simulink model "Complete_Model"  (R2023a)
settings: Solver=VariableStepAuto  StartTime=0.0  StopTime=22  MinStep=5e-5  RelTol=1e-3
values from neighbouring files (symbols this model uses):
  Cbus = 80e-3*n_wt_agg*u^2    (= 1.33333333333)    [param.m]
  D = 1e-3    (= 0.001)    [param.m]
  f = 50    [param.m]
  Fs = Vs * sqrt(2/3)/(2*pi*f)    [param.m]
  fsw = 4e3    (= 4000)    [param.m]
  Idr_rated = sqrt(2)*Is    [param.m]
  J = 127*n_wt_agg    (= 19050)    [param.m]
  ki_id = (wni^2)*Lr*sigma    (= 3.27733001332)    [param.m]
  ki_idg = (wnig^2)*Lg    (= 0.263189450696)    [param.m]
  ki_iq = ki_id    (= 3.27733001332)    [param.m]
  ki_iqg = ki_idg    (= 0.263189450696)    [param.m]
  ki_v = -300000*n_wt_agg    (= -45000000)    [param.m]
  Kopt = ((0.5*rho*pi*(Radius^5)*Cp_max)/(lambda_opt^3))    (= 296454.198186)    [param.m]
  kp_id = (2*wni*sigma*Lr) - Rr    (= 0.00384733333333)    [param.m]
  kp_idg = ((2*wnig*Lg)-Rg)    (= 0.00167538274858)    [param.m]
  kp_iq = kp_id    (= 0.00384733333333)    [param.m]
  kp_iqg = kp_idg    (= 0.00167538274858)    [param.m]
  kp_v = -1000*n_wt_agg    (= -150000)    [param.m]
  Kpg = 1/(1.5*Vr*sqrt(2/3))    [param.m]
  Kqg = -Kpg    [param.m]
  lambda_opt = 7.2    [param.m]
  Lg = 400e-6 /n_wt_agg    (= 2.66666666667e-06)    [param.m]
  Lm = 2.5e-3 /n_wt_agg    (= 1.66666666667e-05)    [param.m]
  Lr = Lm + Lsi    (= 1.72466666667e-05)    [param.m]
  Ls = Lm + Lsi    (= 1.72466666667e-05)    [param.m]
  Lsi = 0.087e-3 /n_wt_agg    (= 5.8e-07)    [param.m]
  N = 100    [param.m]
  n_wt_agg = 150    [param.m]
  p = 2    [param.m]
  Ps = 2e6*n_wt_agg    (= 300000000)    [param.m]
  Radius = 42    [param.m]
  Rg = 20e-6 /n_wt_agg    (= 1.33333333333e-07)    [param.m]
  rho = 1.225    [param.m]
  Rr = 2.9e-3 /n_wt_agg    (= 1.93333333333e-05)    [param.m]
  Rs = 2.6e-3 /n_wt_agg    (= 1.73333333333e-05)    [param.m]
  sigma = 1 - Lm^2 / (Ls*Lr)    (= 0.0661284179513)    [param.m]
  smax = 1/3    (= 0.333333333333)    [param.m]
  tab_lambda = [0.1:0.01:11.8]    [param.m]
  Vbus = 1150/u    (= 3450)    [param.m]
  Vs = 690    [param.m]
undefined symbols (not defined in the file or in its folder): Cp, D1, Delta, DiscIntNeverNeededParam, magnitude
libraries: spsSecondOrderFilterLib x133, spsThreePhaseVIMeasurementLib x58, spsPower3phInstantaneousLib x52, simulink x41, spsThreePhaseTransformerTwoWindingsLib x27, spsUniversalBridgeLib x22, spsPWMGenerator2LevelLib x22, spsSeriesRLCBranchLib x19, spsFirstOrderFilterLib x18, spsThreePhasePISectionLineLib x17, spsGroundLib x17, spsThreePhaseSeriesRLCBranchLib x17
(part 12 of 15)

== system Complete_Model/Equivalent Wind Park/300MW Eq WT10/Power Calculations/angle2 ==
  Vs_mes : Inport
  Demux : Demux  Outputs=2
  Simple PLL : Trigonometry  Operator=atan2
  abc->alpha/beta : Subsystem
  theta_g : Outport
  wires:
  Simple PLL/1 -> theta_g/1  "theta_g"
  Demux/2 -> Simple PLL/1
  Demux/1 -> Simple PLL/2
  abc->alpha/beta/1(alpha/beta) -> Demux/1
  Vs_mes/1 -> abc->alpha/beta/1(abc)

== system Complete_Model/Equivalent Wind Park/300MW Eq WT10/Rotor Side Control/D//Q->abc ==
  note: D/Q = alpha/beta en fait
  D/Q : Inport
  Fcn : Fcn  Expr=u[1]
  Fcn1 : Fcn  Expr=-(u[1]/2) + u[2]*(sqrt(3)/2)
  Fcn2 : Fcn  Expr=-(u[1]/2) - u[2]*(sqrt(3)/2)
  Mux : Mux  Inputs=3
  abc : Outport
  wires:
  Fcn/1 -> Mux/1
  Fcn2/1 -> Mux/3
  Mux/1 -> abc/1
  Fcn1/1 -> Mux/2
  D/Q/1 -> Fcn/1, Fcn1/1, Fcn2/1

== system Complete_Model/Equivalent Wind Park/300MW Eq WT10/Rotor Side Control/D//Q->d//q ==
  D/Q : Inport
  theta : Inport  Port=2
  Fcn : Fcn  Expr=u[1]*cos(u[3]) + u[2]*sin(u[3])
  Fcn1 : Fcn  Expr=-u[1]*sin(u[3]) + u[2]*cos(u[3])
  Mux : Mux  Inputs=2
  Mux1 : Mux  Inputs=2
  d/q : Outport
  wires:
  Fcn1/1 -> Mux/2
  Mux1/1 -> Fcn1/1, Fcn/1
  Fcn/1 -> Mux/1
  theta/1 -> Mux1/2
  D/Q/1 -> Mux1/1
  Mux/1 -> d/q/1

== system Complete_Model/Equivalent Wind Park/300MW Eq WT10/Rotor Side Control/MPPT ==
  omega_m : Inport
  Gain : Gain  Gain=1/N
  Gain1 : Gain  Gain=-Kopt
  Gain2 : Gain  Gain=1/N
  Gain3 : Gain  Gain=n_wt_agg
  Square : Math  Operator=square
  Tm,opt : Outport
  wires:
  Gain3/1 -> Tm,opt/1
  Gain2/1 -> Gain3/1
  Gain1/1 -> Gain2/1
  Square/1 -> Gain1/1
  Gain/1 -> Square/1
  omega_m/1 -> Gain/1

== system Complete_Model/Equivalent Wind Park/300MW Eq WT10/Rotor Side Control/PI id ==
  ref : Inport
  meas : Inport  Port=2
  Discrete-Time Integrator : DiscreteIntegrator  SampleTime=1/fsw  LimitOutput=on  UpperSaturationLimit=Vbus/sqrt(3)  LowerSaturationLimit=-Vbus/sqrt(3)  ICPrevOutput=DiscIntNeverNeededParam  ICPrevScaledInput=DiscIntNeverNeededParam
  Gain : Gain  Gain=kp_id
  Gain1 : Gain  Gain=ki_id
  Saturation : Saturate  UpperLimit=Vbus/sqrt(3)  LowerLimit=-Vbus/sqrt(3)
  Sum : Sum  Inputs=|+-
  Sum1 : Sum  Inputs=|++
  out : Outport
  wires:
  Sum1/1 -> Saturation/1
  Discrete-Time Integrator/1 -> Sum1/2
  Gain1/1 -> Discrete-Time Integrator/1
  Gain/1 -> Sum1/1
  Sum/1 -> Gain/1, Gain1/1
  meas/1 -> Sum/2
  ref/1 -> Sum/1
  Saturation/1 -> out/1

== system Complete_Model/Equivalent Wind Park/300MW Eq WT10/Rotor Side Control/PI iq ==
  ref : Inport
  meas : Inport  Port=2
  Discrete-Time Integrator : DiscreteIntegrator  SampleTime=1/fsw  LimitOutput=on  UpperSaturationLimit=Vbus/sqrt(3)  LowerSaturationLimit=-Vbus/sqrt(3)  ICPrevOutput=DiscIntNeverNeededParam  ICPrevScaledInput=DiscIntNeverNeededParam
  Gain : Gain  Gain=kp_iq
  Gain1 : Gain  Gain=ki_iq
  Saturation : Saturate  UpperLimit=Vbus/sqrt(3)  LowerLimit=-Vbus/sqrt(3)
  Sum : Sum  Inputs=|+-
  Sum1 : Sum  Inputs=|++
  out : Outport
  wires:
  Sum1/1 -> Saturation/1
  Discrete-Time Integrator/1 -> Sum1/2
  Gain1/1 -> Discrete-Time Integrator/1
  Gain/1 -> Sum1/1
  Sum/1 -> Gain/1, Gain1/1
  meas/1 -> Sum/2
  ref/1 -> Sum/1
  Saturation/1 -> out/1

== system Complete_Model/Equivalent Wind Park/300MW Eq WT10/Rotor Side Control/abc->alpha//beta ==
  abc : Inport
  Fcn1 : Fcn  Expr=2/3 * (u[1]-u[2]/2-u[3]/2)
  Fcn2 : Fcn  Expr=2/3 * (u[2] * (sqrt(3)/2) - u[3]*(sqrt(3)/2))
  Mux : Mux  Inputs=2
  alpha/beta : Outport
  wires:
  abc/1 -> Fcn2/1, Fcn1/1
  Mux/1 -> alpha/beta/1
  Fcn1/1 -> Mux/1
  Fcn2/1 -> Mux/2

== system Complete_Model/Equivalent Wind Park/300MW Eq WT10/Rotor Side Control/angle ==
  Vs_mes : Inport
  theta_m : Inport  Port=2
  Constant : Constant  Value=pi/2
  Demux : Demux  Outputs=2
  Simple PLL : Trigonometry  Operator=atan2
  Sum : Sum  Inputs=|+-
  Sum1 : Sum  Inputs=|+-
  abc->alpha/beta : Subsystem
  theta_em = p. theta_m : Gain  Gain=p
  theta_r : Outport
  wires:
  Constant/1 -> Sum/2
  theta_em = p. theta_m/1 -> Sum1/2  "theta_em"
  theta_m/1 -> theta_em = p. theta_m/1
  Sum/1 -> Sum1/1  "theta_s"
  Simple PLL/1 -> Sum/1
  Demux/2 -> Simple PLL/1
  Demux/1 -> Simple PLL/2
  abc->alpha/beta/1(alpha/beta) -> Demux/1
  Vs_mes/1 -> abc->alpha/beta/1(abc)
  Sum1/1 -> theta_r/1

== system Complete_Model/Equivalent Wind Park/300MW Eq WT10/Rotor Side Control/cancellation of coupling terms ==
  note: omega_r
  Vd* : Inport
  Vq* : Inport  Port=2
  omega_m : Inport  Port=3
  Constant1 : Constant  Value=f
  Fcn : Fcn  Expr=u[1]-u[5]*sigma*Lr*u[4]
  Fcn1 : Fcn  Expr=u[2]+u[5]*sigma*Lr*u[3]+u[5]*(Lm/Ls)*u[6]
  Gain : Gain  Gain=2*pi
  Gain1 : Gain  Gain=p
  Mux : Mux  Inputs=6
  Mux1 : Mux  Inputs=2
  Stator Flux : Constant  Value=Fs
  Sum : Sum  Inputs=|+-
  out : Outport
  tag scope: vdr_ref3=global, vqr_ref3=global
  wires:
  Fcn1/1 -> [vqr_ref3], Mux1/2
  Fcn/1 -> [vdr_ref3], Mux1/1
  Mux1/1 -> out/1
  Mux/1 -> Fcn1/1, Fcn/1
  omega_m/1 -> Gain1/1
  Gain1/1 -> Sum/2  "omega_em"
  Gain/1 -> Sum/1  "omega_s"
  Constant1/1 -> Gain/1
  Stator Flux/1 -> Mux/6
  Sum/1 -> Mux/5
  [iqr3] -> Mux/4
  [idr3] -> Mux/3
  Vq*/1 -> Mux/2
  Vd*/1 -> Mux/1

== system Complete_Model/Equivalent Wind Park/300MW Eq WT10/Rotor Side Control/d//q->D//Q ==
  d/q : Inport
  theta : Inport  Port=2
  Fcn : Fcn  Expr=u[1]*cos(u[3])-u[2]*sin(u[3])
  Fcn1 : Fcn  Expr=u[1]*sin(u[3])+u[2]*cos(u[3])
  Mux : Mux  Inputs=2
  Mux1 : Mux  Inputs=2
  D/Q : Outport
  wires:
  Fcn1/1 -> Mux/2
  Mux1/1 -> Fcn1/1, Fcn/1
  Fcn/1 -> Mux/1
  theta/1 -> Mux1/2
  d/q/1 -> Mux1/1
  Mux/1 -> D/Q/1

== system Complete_Model/Equivalent Wind Park/300MW Eq WT11/Grid Side Control/PI  ==
  ref : Inport
  meas : Inport  Port=2
  Discrete Derivative : simulink/Discrete/Discrete Derivative  Commented=on  gainval=1.0  ICPrevScaledInput=0.0  OutMin=[-Ps*smax]  OutMax=[Ps*smax]  OutDataType=fixdt(1, 16)  OutScaling=2^-10  DoSatur=off
  Discrete-Time Integrator : DiscreteIntegrator  SampleTime=1/fsw  LimitOutput=on  UpperSaturationLimit=Ps*smax  LowerSaturationLimit=-Ps*smax  ICPrevOutput=DiscIntNeverNeededParam  ICPrevScaledInput=DiscIntNeverNeededParam
  Gain : Gain  Gain=kp_v
  Gain1 : Gain  Gain=ki_v
  Gain2 : Gain  Commented=on  Gain=ki_v
  PID Controller : slpidlib/PID Controller  Commented=on  Controller=PI  Form=Parallel  TimeDomain=Continuous-time  UseExternalTs=off  SampleTime=-1  IntegratorMethod=Forward Euler  FilterMethod=Forward Euler  ControllerParametersSource=internal  P=kp_v  I=ki_v  UseKiTs=off  D=0  N=100  UseFilter=on  TunerSelectOption=Transfer Function Based (PID Tuner App)  InitialConditionSource=internal  InitialConditionForIntegrator=0  InitialConditionForFilter=0  DifferentiatorICPrevScaledInput=0  ExternalReset=none  TrackingMode=off  Kt=1  LimitOutput=on  SatLimitsSource=internal  UpperSaturationLimit=Ps*smax  LowerSaturationLimit=-Ps*smax  LinearizeAsGain=on  AntiWindupMode=clamping  Kb=1  LimitIntegrator=on  UpperIntegratorSaturationLimit=Ps*smax  LowerIntegratorSaturationLimit=-Ps*smax  POutMin=[]  POutMax=[]  IOutMin=[]  IOutMax=[]  DOutMin=[]  DOutMax=[]  NOutMin=[]  NOutMax=[]  SumOutMin=[]  SumOutMax=[]  SaturationOutMin=[]  SaturationOutMax=[]  PParamMin=[]  PParamMax=[]  IParamMin=[]  IParamMax=[]  DParamMin=[]  DParamMax=[]  NParamMin=[]  NParamMax=[]  KbParamMin=[]  KbParamMax=[]  KtParamMin=[]  KtParamMax=[]  KbOutMin=[]  KbOutMax=[]  KtOutMin=[]  KtOutMax=[]  IntegratorOutMin=[]  IntegratorOutMax=[]  FilterOutMin=[]  FilterOutMax=[]  SumI1OutMin=[]  SumI1OutMax=[]  SumI2OutMin=[]  SumI2OutMax=[]  SumI3OutMin=[]  SumI3OutMax=[]  SumI4OutMin=[]  SumI4OutMax=[]  SumDOutMin=[]  SumDOutMax=[]  DifferentiatorOutMin=[]  DifferentiatorOutMax=[]  FilterDiffOutCoefMin=[]  FilterDiffOutCoefMax=[]  ReciprocalOutMin=[]  ReciprocalOutMax=[]  SumDenOutMin=[]  SumDenOutMax=[]  SumNumOutMin=[]  SumNumOutMax=[]  DivideOutMin=[]  DivideOutMax=[]  UdiffTsProdOutMin=[]  UdiffTsProdOutMax=[]  NTsProdOutMin=[]  NTsProdOutMax=[]  UintegralTsProdOutMin=[]  UintegralTsProdOutMax=[]  UngainTsProdOutMin=[]  UngainTsProdOutMax=[]  ClampingZeroOutDataTypeStr=Inherit: Inherit via back propagation  ClampingZeroOutMin=[]  ClampingZeroOutMax=[]  FilterDenConstantOutDataTypeStr=Inherit: Inherit via back propagation  FilterDenConstantOutMin=[]  FilterDenConstantOutMax=[]  PCopyOutDataTypeStr=Inherit: Inherit via back propagation  PCopyOutMin=[]  PCopyOutMax=[]  NCopyOutDataTypeStr=Inherit: Inherit via back propagation  NCopyOutMin=[]  NCopyOutMax=[]  IntegratorContinuousStateAttributes=''  IntegratorStateMustResolveToSignalObject=off  IntegratorRTWStateStorageClass=Auto  FilterContinuousStateAttributes=''  FilterStateMustResolveToSignalObject=off  FilterRTWStateStorageClass=Auto  ParallelPVariant=InternalParameters  IdealPVariant=Disabled  IVariant=InternalParameters  DVariant=Disabled  IntegratorVariant=Continuous  SatVariant=Enabled  AWVariant=ContClampingParallel  PCopyVariant=Disabled  TRVariant=Disabled  FdbkBlocksVariant=Forward  IdealPFdbkVariant=Disabled  SatFdbkVariant=Disabled  DerivativeFilterVariant=Disabled  NVariant=Disabled  NCopyVariant=Disabled_SignalSpec  FilterICVariant=Disabled  IntegratorICVariant=InternalICs  ExternalResetVariant=Disabled  TRSumVariant=Passthrough  SumFdbkVariant=Disabled  SumVariant=Sum_PI  TsampFilterVariant=InternalTs  TsampNgainVariant=Passthrough  TsampIntegralVariant=Disabled_SignalSpec  ExternalSaturationOutDataTypeStr=Inherit: Same as second input
  Saturation : Saturate  UpperLimit=Ps*smax  LowerLimit=-Ps*smax
  Sum : Sum  Inputs=|+-
  Sum1 : Sum  Inputs=|+++
  out : Outport
  wires:
  Discrete Derivative/1 -> Sum1/3
  Gain2/1 -> Discrete Derivative/1
  Sum1/1 -> Saturation/1
  Discrete-Time Integrator/1 -> Sum1/2
  Gain1/1 -> Discrete-Time Integrator/1
  Gain/1 -> Sum1/1
  Sum/1 -> Gain/1, Gain1/1, Gain2/1
  meas/1 -> Sum/2
  ref/1 -> Sum/1
  Saturation/1 -> out/1

== system Complete_Model/Equivalent Wind Park/300MW Eq WT11/Grid Side Control/PI id ==
  ref : Inport
  meas : Inport  Port=2
  Discrete-Time Integrator : DiscreteIntegrator  SampleTime=1/fsw  LimitOutput=on  UpperSaturationLimit=Vbus/sqrt(3)  LowerSaturationLimit=-Vbus/sqrt(3)  ICPrevOutput=DiscIntNeverNeededParam  ICPrevScaledInput=DiscIntNeverNeededParam
  Gain : Gain  Gain=kp_idg
  Gain1 : Gain  Gain=ki_idg
  Saturation : Saturate  UpperLimit=Vbus/sqrt(3)  LowerLimit=-Vbus/sqrt(3)
  Sum : Sum  Inputs=|+-
  Sum1 : Sum  Inputs=|++
  out : Outport
  wires:
  Sum1/1 -> Saturation/1
  Discrete-Time Integrator/1 -> Sum1/2
  Gain1/1 -> Discrete-Time Integrator/1
  Gain/1 -> Sum1/1
  Sum/1 -> Gain/1, Gain1/1
  meas/1 -> Sum/2
  ref/1 -> Sum/1
  Saturation/1 -> out/1

== system Complete_Model/Equivalent Wind Park/300MW Eq WT11/Grid Side Control/PI iq ==
  ref : Inport
  meas : Inport  Port=2
  Discrete-Time Integrator : DiscreteIntegrator  SampleTime=1/fsw  LimitOutput=on  UpperSaturationLimit=Vbus/sqrt(3)  LowerSaturationLimit=-Vbus/sqrt(3)  ICPrevOutput=DiscIntNeverNeededParam  ICPrevScaledInput=DiscIntNeverNeededParam
  Gain : Gain  Gain=kp_iqg
  Gain1 : Gain  Gain=ki_iqg
  Saturation : Saturate  UpperLimit=Vbus/sqrt(3)  LowerLimit=-Vbus/sqrt(3)
  Sum : Sum  Inputs=|+-
  Sum1 : Sum  Inputs=|++
  out : Outport
  wires:
  Sum1/1 -> Saturation/1
  Discrete-Time Integrator/1 -> Sum1/2
  Gain1/1 -> Discrete-Time Integrator/1
  Gain/1 -> Sum1/1
  Sum/1 -> Gain/1, Gain1/1
  meas/1 -> Sum/2
  ref/1 -> Sum/1
  Saturation/1 -> out/1

== system Complete_Model/Equivalent Wind Park/300MW Eq WT11/Grid Side Control/abc->alpha//beta ==
  abc : Inport
  Fcn1 : Fcn  Expr=2/3 * (u[1]-u[2]/2-u[3]/2)
  Fcn2 : Fcn  Expr=2/3 * (u[2] * (sqrt(3)/2) - u[3]*(sqrt(3)/2))
  Mux : Mux  Inputs=2
  alpha/beta : Outport
  wires:
  abc/1 -> Fcn2/1, Fcn1/1
  Mux/1 -> alpha/beta/1
  Fcn1/1 -> Mux/1
  Fcn2/1 -> Mux/2

== system Complete_Model/Equivalent Wind Park/300MW Eq WT11/Grid Side Control/abc->alpha//beta1 ==
  abc : Inport
  Fcn1 : Fcn  Expr=2/3 * (u[1]-u[2]/2-u[3]/2)
  Fcn2 : Fcn  Expr=2/3 * (u[2] * (sqrt(3)/2) - u[3]*(sqrt(3)/2))
  Mux : Mux  Inputs=2
  alpha/beta : Outport
  wires:
  abc/1 -> Fcn2/1, Fcn1/1
  Mux/1 -> alpha/beta/1
  Fcn1/1 -> Mux/1
  Fcn2/1 -> Mux/2

== system Complete_Model/Equivalent Wind Park/300MW Eq WT11/Grid Side Control/alpha//beta->abc ==
  note: D/Q = alpha/beta en fait
  D/Q : Inport
  Fcn : Fcn  Expr=u[1]
  Fcn1 : Fcn  Expr=-(u[1]/2) + u[2]*(sqrt(3)/2)
  Fcn2 : Fcn  Expr=-(u[1]/2) - u[2]*(sqrt(3)/2)
  Mux : Mux  Inputs=3
  abc : Outport
  wires:
  Fcn/1 -> Mux/1
  Fcn2/1 -> Mux/3
  Mux/1 -> abc/1
  Fcn1/1 -> Mux/2
  D/Q/1 -> Fcn/1, Fcn1/1, Fcn2/1

== system Complete_Model/Equivalent Wind Park/300MW Eq WT11/Grid Side Control/alpha//beta->d//q ==
  D/Q : Inport
  theta : Inport  Port=2
  Fcn : Fcn  Expr=u[1]*cos(u[3]) + u[2]*sin(u[3])
  Fcn1 : Fcn  Expr=-u[1]*sin(u[3]) + u[2]*cos(u[3])
  Mux : Mux  Inputs=2
  Mux1 : Mux  Inputs=2
  d/q : Outport
  wires:
  Fcn1/1 -> Mux/2
  Mux1/1 -> Fcn1/1, Fcn/1
  Fcn/1 -> Mux/1
  theta/1 -> Mux1/2
  D/Q/1 -> Mux1/1
  Mux/1 -> d/q/1

== system Complete_Model/Equivalent Wind Park/300MW Eq WT11/Grid Side Control/alpha//beta->d//q1 ==
  D/Q : Inport
  theta : Inport  Port=2
  Fcn : Fcn  Expr=u[1]*cos(u[3]) + u[2]*sin(u[3])
  Fcn1 : Fcn  Expr=-u[1]*sin(u[3]) + u[2]*cos(u[3])
  Mux : Mux  Inputs=2
  Mux1 : Mux  Inputs=2
  d/q : Outport
  wires:
  Mux/1 -> d/q/1
  D/Q/1 -> Mux1/1
  theta/1 -> Mux1/2
  Fcn/1 -> Mux/1
  Mux1/1 -> Fcn/1, Fcn1/1
  Fcn1/1 -> Mux/2

== system Complete_Model/Equivalent Wind Park/300MW Eq WT11/Grid Side Control/angle2 ==
  Vs_mes : Inport
  Demux : Demux  Outputs=2
  Simple PLL : Trigonometry  Operator=atan2
  abc->alpha/beta : Subsystem
  theta_g : Outport
  wires:
  Simple PLL/1 -> theta_g/1  "theta_g"
  Demux/2 -> Simple PLL/1
  Demux/1 -> Simple PLL/2
  abc->alpha/beta/1(alpha/beta) -> Demux/1
  Vs_mes/1 -> abc->alpha/beta/1(abc)

== system Complete_Model/Equivalent Wind Park/300MW Eq WT11/Grid Side Control/cancellation of coupling terms ==
  Vd* : Inport
  Vq* : Inport  Port=2
  Fcn : Fcn  Expr=u[1]-u[4]*Lg*(2*pi*f)
  Fcn1 : Fcn  Expr=u[2]+u[3]*Lg*(2*pi*f)
  Mux : Mux
  Mux1 : Mux  Inputs=2
  out : Outport
  tag scope: vdg_ref22=global, vqg_ref22=global
  wires:
  Fcn1/1 -> Mux1/2, [vqg_ref22]
  Fcn/1 -> Mux1/1, [vdg_ref22]
  Mux1/1 -> out/1
  Mux/1 -> Fcn1/1, Fcn/1
  [iqg22] -> Mux/4
  [idg22] -> Mux/3
  Vq*/1 -> Mux/2
  Vd*/1 -> Mux/1

== system Complete_Model/Equivalent Wind Park/300MW Eq WT11/Grid Side Control/d//q->alpha//beta ==
  d/q : Inport
  theta : Inport  Port=2
  Fcn : Fcn  Expr=u[1]*cos(u[3])-u[2]*sin(u[3])
  Fcn1 : Fcn  Expr=u[1]*sin(u[3])+u[2]*cos(u[3])
  Mux : Mux  Inputs=2
  Mux1 : Mux  Inputs=2
  D/Q : Outport
  wires:
  Fcn1/1 -> Mux/2
  Mux1/1 -> Fcn1/1, Fcn/1
  Fcn/1 -> Mux/1
  theta/1 -> Mux1/2
  d/q/1 -> Mux1/1
  Mux/1 -> D/Q/1

== system Complete_Model/Equivalent Wind Park/300MW Eq WT11/Power Calculations/Subsystem ==
  abc : Inport
  Fcn1 : Fcn  Expr=2/3 * (u[1]-u[2]/2-u[3]/2)
  Fcn2 : Fcn  Expr=2/3 * (u[2] * (sqrt(3)/2) - u[3]*(sqrt(3)/2))
  Mux : Mux  Inputs=2
  alpha/beta : Outport
  wires:
  Mux/1 -> alpha/beta/1
  Fcn1/1 -> Mux/1
  abc/1 -> Fcn2/1, Fcn1/1
  Fcn2/1 -> Mux/2

== system Complete_Model/Equivalent Wind Park/300MW Eq WT11/Power Calculations/Subsystem1 ==
  abc : Inport
  Fcn1 : Fcn  Expr=2/3 * (u[1]-u[2]/2-u[3]/2)
  Fcn2 : Fcn  Expr=2/3 * (u[2] * (sqrt(3)/2) - u[3]*(sqrt(3)/2))
  Mux : Mux  Inputs=2
  alpha/beta : Outport
  wires:
  Fcn2/1 -> Mux/2
  abc/1 -> Fcn1/1, Fcn2/1
  Fcn1/1 -> Mux/1
  Mux/1 -> alpha/beta/1

== system Complete_Model/Equivalent Wind Park/300MW Eq WT11/Power Calculations/Subsystem2 ==
  abc : Inport
  Fcn1 : Fcn  Expr=2/3 * (u[1]-u[2]/2-u[3]/2)
  Fcn2 : Fcn  Expr=2/3 * (u[2] * (sqrt(3)/2) - u[3]*(sqrt(3)/2))
  Mux : Mux  Inputs=2
  alpha/beta : Outport
  wires:
  Fcn2/1 -> Mux/2
  abc/1 -> Fcn1/1, Fcn2/1
  Fcn1/1 -> Mux/1
  Mux/1 -> alpha/beta/1

== system Complete_Model/Equivalent Wind Park/300MW Eq WT11/Power Calculations/Subsystem3 ==
  abc : Inport
  Fcn1 : Fcn  Expr=2/3 * (u[1]-u[2]/2-u[3]/2)
  Fcn2 : Fcn  Expr=2/3 * (u[2] * (sqrt(3)/2) - u[3]*(sqrt(3)/2))
  Mux : Mux  Inputs=2
  alpha/beta : Outport
  wires:
  Mux/1 -> alpha/beta/1
  Fcn1/1 -> Mux/1
  abc/1 -> Fcn2/1, Fcn1/1
  Fcn2/1 -> Mux/2

== system Complete_Model/Equivalent Wind Park/300MW Eq WT11/Power Calculations/alpha//beta->d//q ==
  D/Q : Inport
  theta : Inport  Port=2
  Fcn : Fcn  Expr=u[1]*cos(u[3]) + u[2]*sin(u[3])
  Fcn1 : Fcn  Expr=-u[1]*sin(u[3]) + u[2]*cos(u[3])
  Mux : Mux  Inputs=2
  Mux1 : Mux  Inputs=2
  d/q : Outport
  wires:
  Fcn1/1 -> Mux/2
  Mux1/1 -> Fcn1/1, Fcn/1
  Fcn/1 -> Mux/1
  theta/1 -> Mux1/2
  D/Q/1 -> Mux1/1
  Mux/1 -> d/q/1

== system Complete_Model/Equivalent Wind Park/300MW Eq WT11/Power Calculations/alpha//beta->d//q1 ==
  D/Q : Inport
  theta : Inport  Port=2
  Fcn : Fcn  Expr=u[1]*cos(u[3]) + u[2]*sin(u[3])
  Fcn1 : Fcn  Expr=-u[1]*sin(u[3]) + u[2]*cos(u[3])
  Mux : Mux  Inputs=2
  Mux1 : Mux  Inputs=2
  d/q : Outport
  wires:
  Mux/1 -> d/q/1
  D/Q/1 -> Mux1/1
  theta/1 -> Mux1/2
  Fcn/1 -> Mux/1
  Mux1/1 -> Fcn/1, Fcn1/1
  Fcn1/1 -> Mux/2

== system Complete_Model/Equivalent Wind Park/300MW Eq WT11/Power Calculations/alpha//beta->d//q2 ==
  D/Q : Inport
  theta : Inport  Port=2
  Fcn : Fcn  Expr=u[1]*cos(u[3]) + u[2]*sin(u[3])
  Fcn1 : Fcn  Expr=-u[1]*sin(u[3]) + u[2]*cos(u[3])
  Mux : Mux  Inputs=2
  Mux1 : Mux  Inputs=2
  d/q : Outport
  wires:
  Mux/1 -> d/q/1
  D/Q/1 -> Mux1/1
  theta/1 -> Mux1/2
  Fcn/1 -> Mux/1
  Mux1/1 -> Fcn/1, Fcn1/1
  Fcn1/1 -> Mux/2

== system Complete_Model/Equivalent Wind Park/300MW Eq WT11/Power Calculations/alpha//beta->d//q3 ==
  D/Q : Inport
  theta : Inport  Port=2
  Fcn : Fcn  Expr=u[1]*cos(u[3]) + u[2]*sin(u[3])
  Fcn1 : Fcn  Expr=-u[1]*sin(u[3]) + u[2]*cos(u[3])
  Mux : Mux  Inputs=2
  Mux1 : Mux  Inputs=2
  d/q : Outport
  wires:
  Fcn1/1 -> Mux/2
  Mux1/1 -> Fcn1/1, Fcn/1
  Fcn/1 -> Mux/1
  theta/1 -> Mux1/2
  D/Q/1 -> Mux1/1
  Mux/1 -> d/q/1

== system Complete_Model/Equivalent Wind Park/300MW Eq WT11/Power Calculations/angle1 ==
  Vs_mes : Inport
  Demux : Demux  Outputs=2
  Simple PLL : Trigonometry  Operator=atan2
  abc->alpha/beta : Subsystem
  theta_g : Outport
  wires:
  Vs_mes/1 -> abc->alpha/beta/1(abc)
  abc->alpha/beta/1(alpha/beta) -> Demux/1
  Demux/1 -> Simple PLL/2
  Demux/2 -> Simple PLL/1
  Simple PLL/1 -> theta_g/1  "theta_g"

== system Complete_Model/Equivalent Wind Park/300MW Eq WT11/Power Calculations/angle2 ==
  Vs_mes : Inport
  Demux : Demux  Outputs=2
  Simple PLL : Trigonometry  Operator=atan2
  abc->alpha/beta : Subsystem
  theta_g : Outport
  wires:
  Simple PLL/1 -> theta_g/1  "theta_g"
  Demux/2 -> Simple PLL/1
  Demux/1 -> Simple PLL/2
  abc->alpha/beta/1(alpha/beta) -> Demux/1
  Vs_mes/1 -> abc->alpha/beta/1(abc)

== system Complete_Model/Equivalent Wind Park/300MW Eq WT11/Rotor Side Control/D//Q->abc ==
  note: D/Q = alpha/beta en fait
  D/Q : Inport
  Fcn : Fcn  Expr=u[1]
  Fcn1 : Fcn  Expr=-(u[1]/2) + u[2]*(sqrt(3)/2)
  Fcn2 : Fcn  Expr=-(u[1]/2) - u[2]*(sqrt(3)/2)
  Mux : Mux  Inputs=3
  abc : Outport
  wires:
  Fcn/1 -> Mux/1
  Fcn2/1 -> Mux/3
  Mux/1 -> abc/1
  Fcn1/1 -> Mux/2
  D/Q/1 -> Fcn/1, Fcn1/1, Fcn2/1

== system Complete_Model/Equivalent Wind Park/300MW Eq WT11/Rotor Side Control/D//Q->d//q ==
  D/Q : Inport
  theta : Inport  Port=2
  Fcn : Fcn  Expr=u[1]*cos(u[3]) + u[2]*sin(u[3])
  Fcn1 : Fcn  Expr=-u[1]*sin(u[3]) + u[2]*cos(u[3])
  Mux : Mux  Inputs=2
  Mux1 : Mux  Inputs=2
  d/q : Outport
  wires:
  Fcn1/1 -> Mux/2
  Mux1/1 -> Fcn1/1, Fcn/1
  Fcn/1 -> Mux/1
  theta/1 -> Mux1/2
  D/Q/1 -> Mux1/1
  Mux/1 -> d/q/1

== system Complete_Model/Equivalent Wind Park/300MW Eq WT11/Rotor Side Control/MPPT ==
  omega_m : Inport
  Gain : Gain  Gain=1/N
  Gain1 : Gain  Gain=-Kopt
  Gain2 : Gain  Gain=1/N
  Gain3 : Gain  Gain=n_wt_agg
  Square : Math  Operator=square
  Tm,opt : Outport
  wires:
  Gain3/1 -> Tm,opt/1
  Gain2/1 -> Gain3/1
  Gain1/1 -> Gain2/1
  Square/1 -> Gain1/1
  Gain/1 -> Square/1
  omega_m/1 -> Gain/1

== system Complete_Model/Equivalent Wind Park/300MW Eq WT11/Rotor Side Control/PI id ==
  ref : Inport
  meas : Inport  Port=2
  Discrete-Time Integrator : DiscreteIntegrator  SampleTime=1/fsw  LimitOutput=on  UpperSaturationLimit=Vbus/sqrt(3)  LowerSaturationLimit=-Vbus/sqrt(3)  ICPrevOutput=DiscIntNeverNeededParam  ICPrevScaledInput=DiscIntNeverNeededParam
  Gain : Gain  Gain=kp_id
  Gain1 : Gain  Gain=ki_id
  Saturation : Saturate  UpperLimit=Vbus/sqrt(3)  LowerLimit=-Vbus/sqrt(3)
  Sum : Sum  Inputs=|+-
  Sum1 : Sum  Inputs=|++
  out : Outport
  wires:
  Sum1/1 -> Saturation/1
  Discrete-Time Integrator/1 -> Sum1/2
  Gain1/1 -> Discrete-Time Integrator/1
  Gain/1 -> Sum1/1
  Sum/1 -> Gain/1, Gain1/1
  meas/1 -> Sum/2
  ref/1 -> Sum/1
  Saturation/1 -> out/1

== system Complete_Model/Equivalent Wind Park/300MW Eq WT11/Rotor Side Control/PI iq ==
  ref : Inport
  meas : Inport  Port=2
  Discrete-Time Integrator : DiscreteIntegrator  SampleTime=1/fsw  LimitOutput=on  UpperSaturationLimit=Vbus/sqrt(3)  LowerSaturationLimit=-Vbus/sqrt(3)  ICPrevOutput=DiscIntNeverNeededParam  ICPrevScaledInput=DiscIntNeverNeededParam
  Gain : Gain  Gain=kp_iq
  Gain1 : Gain  Gain=ki_iq
  Saturation : Saturate  UpperLimit=Vbus/sqrt(3)  LowerLimit=-Vbus/sqrt(3)
  Sum : Sum  Inputs=|+-
  Sum1 : Sum  Inputs=|++
  out : Outport
  wires:
  Sum1/1 -> Saturation/1
  Discrete-Time Integrator/1 -> Sum1/2
  Gain1/1 -> Discrete-Time Integrator/1
  Gain/1 -> Sum1/1
  Sum/1 -> Gain/1, Gain1/1
  meas/1 -> Sum/2
  ref/1 -> Sum/1
  Saturation/1 -> out/1

== system Complete_Model/Equivalent Wind Park/300MW Eq WT11/Rotor Side Control/abc->alpha//beta ==
  abc : Inport
  Fcn1 : Fcn  Expr=2/3 * (u[1]-u[2]/2-u[3]/2)
  Fcn2 : Fcn  Expr=2/3 * (u[2] * (sqrt(3)/2) - u[3]*(sqrt(3)/2))
  Mux : Mux  Inputs=2
  alpha/beta : Outport
  wires:
  abc/1 -> Fcn2/1, Fcn1/1
  Mux/1 -> alpha/beta/1
  Fcn1/1 -> Mux/1
  Fcn2/1 -> Mux/2
